simulink model "gmrt_001_intial"  (Simulink 7.5) (continued)
== system gmrt_001_intial == (continued)
      | %else
      | %    set_param(gcb, 'MaskEnables', {'on','on','on','on','on','on','on','on','on','on','on','on','on','on','on','on'});
      | %end
      | 
      | |%specify_mult = get_param(gcb,'specify_mult');
      | %if( strcmp(specify_mult,'off')),
      | %    set_param(gcb, 'MaskEnables', {'on','on','on','on','on','on','on','on','on','on','on','on','on','on','on','off'});
      | %else
      | %    set_param(gcb, 'MaskEnables', {'on','on','on','on','on','on','on','on','on','on','on','on','on','on','on','on'});
      | %end
  pfb_fir_mux3 : Subsystem  AncestorBlock=casper_library_pfbs/pfb_fir_mux  mask(pfb_fir_mux){PFBSize=10, mux_inputs=2, TotalTaps=4, WindowType=hamming, n_inputs=0, MakeBiplex=1, BitWidthIn=12, BitWidthOut=18, CoeffBitWidth=18, CoeffDistMem=0, add_latency=1, mult_latency=2, bram_latency=3, quantization=Round  (unbiased: +/- Inf), fwidth=1, specify_mult=off, mult_spec=[2                                      2]}
      mask prompts: PFBSize "Size of PFB: (2^? pnts)"; mux_inputs "Signals per input (2^?)"; TotalTaps "Total Number of Taps:"; WindowType "Windowing Function:"; n_inputs "Number of Simultaneous Inputs: (2^?)"; MakeBiplex "Make Biplex"; BitWidthIn "Input Bitwidth:"; BitWidthOut "Output Bitwidth:"; CoeffBitWidth "Coefficient Bitwidth:"; CoeffDistMem "Use Distributed Memory for Coeffs"; add_latency "Add Latency"; mult_latency "Mult Latency"; bram_latency "BRAM Latency"; quantization "Quantization Behavior"; fwidth "Bin Width Scaling (normal=1)"; specify_mult "Specify multiplier use (behavioral if not specified)"; mult_spec "Multiplier specification (0=core, 1=embedded, 2=behavioural) (left=1st tap)"
      mask init: same as gmrt_001_intial/pfb_fir_mux1
      settings: AttributesFormatString=taps=4, add_latency=1
      mask Help: eval('xlWeb(''http://casper.berkeley.edu/wiki/Pfb_fir'')')
      mask StyleString: edit,edit,edit,popup(bartlett|barthannwin|blackman|blackmanharris|bohamwin|chebwin|flattopwin|gausswin|hamming|hann|kaiser|nuttallwin|parzenwin|rectwin|tukeywin|triang|userwindow),edit,edit,edit,edit,edit,edit,edit,edit,edit,popup(Truncate|Round  (unbiased: +/- Inf)|Round  (unbiased: Even Values)),edit,checkbox,edit
      mask CallbackString:
      | ||%num_taps = eval(get_param(gcb, 'TotalTaps'));
      | %old_vec = eval(get_param(gcb, 'mult_spec'));
      | 
      | %vec = 2.*ones(1,num_taps);
      | %old_size = length(old_vec);
      | 
      | %if(old_size <= num_taps),
      | %   new_size = old_size;
      | %else,
      | %    new_size = num_taps;
      | %end
      | 
      | %for n = 1:new_size, vec(n) = old_vec(n); end
      | %set_param(gcb, 'mult_spec', mat2str(vec));||||||||||||%num_taps = eval(get_param(gcb, 'TotalTaps'));
      | %old_vec = eval(get_param(gcb, 'mult_spec'));
      | 
      | %vec = 2.*ones(1,num_taps);
      | %old_size = length(old_vec);
      | 
      | %if(old_size <= num_taps),
      | %    new_size = old_size;
      | %else,
      | %    new_size = num_taps;
      | %end
      | 
      | %for n = 1:new_size, vec(n) = old_vec(n); end
      | %set_param(gcb, 'mult_spec', mat2str(vec));|%specify_mult = get_param(gcb,'specify_mult');
      | %if( strcmp(specify_mult,'off')),
      | %    set_param(gcb, 'MaskEnables', {'on','on','on','on','on','on','on','on','on','on','on','on','on','on','on','off'});
      | %else
      | %    set_param(gcb, 'MaskEnables', {'on','on','on','on','on','on','on','on','on','on','on','on','on','on','on','on'});
      | %end
      | 
      | |%specify_mult = get_param(gcb,'specify_mult');
      | %if( strcmp(specify_mult,'off')),
      | %    set_param(gcb, 'MaskEnables', {'on','on','on','on','on','on','on','on','on','on','on','on','on','on','on','off'});
      | %else
      | %    set_param(gcb, 'MaskEnables', {'on','on','on','on','on','on','on','on','on','on','on','on','on','on','on','on'});
      | %end
  pfb_fir_mux4 : Subsystem  AncestorBlock=casper_library_pfbs/pfb_fir_mux  mask(pfb_fir_mux){PFBSize=10, mux_inputs=2, TotalTaps=4, WindowType=hamming, n_inputs=0, MakeBiplex=1, BitWidthIn=12, BitWidthOut=18, CoeffBitWidth=18, CoeffDistMem=0, add_latency=1, mult_latency=2, bram_latency=3, quantization=Round  (unbiased: +/- Inf), fwidth=1, specify_mult=off, mult_spec=[2                                      2]}
      mask prompts: PFBSize "Size of PFB: (2^? pnts)"; mux_inputs "Signals per input (2^?)"; TotalTaps "Total Number of Taps:"; WindowType "Windowing Function:"; n_inputs "Number of Simultaneous Inputs: (2^?)"; MakeBiplex "Make Biplex"; BitWidthIn "Input Bitwidth:"; BitWidthOut "Output Bitwidth:"; CoeffBitWidth "Coefficient Bitwidth:"; CoeffDistMem "Use Distributed Memory for Coeffs"; add_latency "Add Latency"; mult_latency "Mult Latency"; bram_latency "BRAM Latency"; quantization "Quantization Behavior"; fwidth "Bin Width Scaling (normal=1)"; specify_mult "Specify multiplier use (behavioral if not specified)"; mult_spec "Multiplier specification (0=core, 1=embedded, 2=behavioural) (left=1st tap)"
      mask init: same as gmrt_001_intial/pfb_fir_mux1
      settings: AttributesFormatString=taps=4, add_latency=1
      mask Help: eval('xlWeb(''http://casper.berkeley.edu/wiki/Pfb_fir'')')
      mask StyleString: edit,edit,edit,popup(bartlett|barthannwin|blackman|blackmanharris|bohamwin|chebwin|flattopwin|gausswin|hamming|hann|kaiser|nuttallwin|parzenwin|rectwin|tukeywin|triang|userwindow),edit,edit,edit,edit,edit,edit,edit,edit,edit,popup(Truncate|Round  (unbiased: +/- Inf)|Round  (unbiased: Even Values)),edit,checkbox,edit
      mask CallbackString:
      | ||%num_taps = eval(get_param(gcb, 'TotalTaps'));
      | %old_vec = eval(get_param(gcb, 'mult_spec'));
      | 
      | %vec = 2.*ones(1,num_taps);
      | %old_size = length(old_vec);
      | 
      | %if(old_size <= num_taps),
      | %   new_size = old_size;
      | %else,
      | %    new_size = num_taps;
      | %end
      | 
      | %for n = 1:new_size, vec(n) = old_vec(n); end
      | %set_param(gcb, 'mult_spec', mat2str(vec));||||||||||||%num_taps = eval(get_param(gcb, 'TotalTaps'));
      | %old_vec = eval(get_param(gcb, 'mult_spec'));
      | 
      | %vec = 2.*ones(1,num_taps);
      | %old_size = length(old_vec);
      | 
      | %if(old_size <= num_taps),
      | %    new_size = old_size;
      | %else,
      | %    new_size = num_taps;
      | %end
      | 
      | %for n = 1:new_size, vec(n) = old_vec(n); end
      | %set_param(gcb, 'mult_spec', mat2str(vec));|%specify_mult = get_param(gcb,'specify_mult');
      | %if( strcmp(specify_mult,'off')),
      | %    set_param(gcb, 'MaskEnables', {'on','on','on','on','on','on','on','on','on','on','on','on','on','on','on','off'});
      | %else
      | %    set_param(gcb, 'MaskEnables', {'on','on','on','on','on','on','on','on','on','on','on','on','on','on','on','on'});
      | %end
      | 
      | |%specify_mult = get_param(gcb,'specify_mult');
      | %if( strcmp(specify_mult,'off')),
      | %    set_param(gcb, 'MaskEnables', {'on','on','on','on','on','on','on','on','on','on','on','on','on','on','on','off'});
      | %else
      | %    set_param(gcb, 'MaskEnables', {'on','on','on','on','on','on','on','on','on','on','on','on','on','on','on','on'});
      | %end
  phase_EQ1 : Subsystem  mask{N=10+5 - 2, bram_lat=2, mult_lat=3, add_lat=2, chans_per_coeff=2}
      mask prompts: N "number of coefficients (2^?)"; bram_lat "bram latency"; mult_lat "multiplier latency"; add_lat "adder latency"; chans_per_coeff "channels per coeff (2^?)"
  pipeline : Subsystem  AncestorBlock=casper_library/Delays/pipeline  mask(pipeline){latency=1}
      mask help:
      | An explicitly laid-out delay line to avoid mapping to SRL16s
      | for use in pipelining to help achieve timing closure.
      mask init:
      | pipeline_init(gcb, 'latency', latency);
      mask Display:
      | port_label('input', 1, 'd');
      | port_label('output', 1, 'q');
      | disp(['Z^-', num2str(latency)]);
  pipeline1 : Subsystem  AncestorBlock=casper_library/Delays/pipeline  mask(pipeline){latency=2}
      mask help:
      | An explicitly laid-out delay line to avoid mapping to SRL16s
      | for use in pipelining to help achieve timing closure.
      mask init:
      | pipeline_init(gcb, 'latency', latency);
      mask Display:
      | port_label('input', 1, 'd');
      | port_label('output', 1, 'q');
      | disp(['Z^-', num2str(latency)]);
  pipeline10 : Subsystem  AncestorBlock=casper_library/Delays/pipeline  mask(pipeline){latency=1}
      mask help:
      | An explicitly laid-out delay line to avoid mapping to SRL16s
      | for use in pipelining to help achieve timing closure.
      mask init:
      | pipeline_init(gcb, 'latency', latency);
      mask Display:
      | port_label('input', 1, 'd');
      | port_label('output', 1, 'q');
      | disp(['Z^-', num2str(latency)]);
  pipeline11 : Subsystem  AncestorBlock=casper_library/Delays/pipeline  mask(pipeline){latency=1}
      mask help:
      | An explicitly laid-out delay line to avoid mapping to SRL16s
      | for use in pipelining to help achieve timing closure.
      mask init:
      | pipeline_init(gcb, 'latency', latency);
      mask Display:
      | port_label('input', 1, 'd');
      | port_label('output', 1, 'q');
      | disp(['Z^-', num2str(latency)]);
  pipeline12 : Subsystem  AncestorBlock=casper_library/Delays/pipeline  mask(pipeline){latency=1}
      mask help:
      | An explicitly laid-out delay line to avoid mapping to SRL16s
      | for use in pipelining to help achieve timing closure.
      mask init:
      | pipeline_init(gcb, 'latency', latency);
      mask Display:
      | port_label('input', 1, 'd');
      | port_label('output', 1, 'q');
      | disp(['Z^-', num2str(latency)]);
  pipeline13 : Subsystem  AncestorBlock=casper_library/Delays/pipeline  mask(pipeline){latency=1}
      mask help:
      | An explicitly laid-out delay line to avoid mapping to SRL16s
      | for use in pipelining to help achieve timing closure.
      mask init:
      | pipeline_init(gcb, 'latency', latency);
      mask Display:
      | port_label('input', 1, 'd');
      | port_label('output', 1, 'q');
      | disp(['Z^-', num2str(latency)]);
  pipeline14 : Subsystem  AncestorBlock=casper_library/Delays/pipeline  mask(pipeline){latency=1}
      mask help:
      | An explicitly laid-out delay line to avoid mapping to SRL16s
      | for use in pipelining to help achieve timing closure.
      mask init:
      | pipeline_init(gcb, 'latency', latency);
      mask Display:
      | port_label('input', 1, 'd');
      | port_label('output', 1, 'q');
      | disp(['Z^-', num2str(latency)]);
  pipeline15 : Subsystem  AncestorBlock=casper_library/Delays/pipeline  mask(pipeline){latency=1}
      mask help:
      | An explicitly laid-out delay line to avoid mapping to SRL16s
      | for use in pipelining to help achieve timing closure.
      mask init:
      | pipeline_init(gcb, 'latency', latency);
      mask Display:
      | port_label('input', 1, 'd');
      | port_label('output', 1, 'q');
      | disp(['Z^-', num2str(latency)]);
  pipeline16 : Subsystem  AncestorBlock=casper_library/Delays/pipeline  mask(pipeline){latency=1}
      mask help:
      | An explicitly laid-out delay line to avoid mapping to SRL16s
      | for use in pipelining to help achieve timing closure.
      mask init:
      | pipeline_init(gcb, 'latency', latency);
      mask Display:
      | port_label('input', 1, 'd');
      | port_label('output', 1, 'q');
      | disp(['Z^-', num2str(latency)]);
  pipeline17 : Subsystem  AncestorBlock=casper_library/Delays/pipeline  mask(pipeline){latency=2}
      mask help:
      | An explicitly laid-out delay line to avoid mapping to SRL16s
      | for use in pipelining to help achieve timing closure.
      mask init:
      | pipeline_init(gcb, 'latency', latency);
      mask Display:
      | port_label('input', 1, 'd');
      | port_label('output', 1, 'q');
      | disp(['Z^-', num2str(latency)]);
  pipeline24 : Subsystem  AncestorBlock=casper_library/Delays/pipeline  mask(pipeline){latency=1}
      mask help:
      | An explicitly laid-out delay line to avoid mapping to SRL16s
      | for use in pipelining to help achieve timing closure.
      mask init:
      | pipeline_init(gcb, 'latency', latency);
      mask Display:
      | port_label('input', 1, 'd');
      | port_label('output', 1, 'q');
      | disp(['Z^-', num2str(latency)]);
  pipeline25 : Subsystem  AncestorBlock=casper_library/Delays/pipeline  mask(pipeline){latency=1}
      mask help:
      | An explicitly laid-out delay line to avoid mapping to SRL16s
      | for use in pipelining to help achieve timing closure.
      mask init:
      | pipeline_init(gcb, 'latency', latency);
      mask Display:
      | port_label('input', 1, 'd');
      | port_label('output', 1, 'q');
      | disp(['Z^-', num2str(latency)]);
  pipeline26 : Subsystem  AncestorBlock=casper_library/Delays/pipeline  mask(pipeline){latency=1}
      mask help:
      | An explicitly laid-out delay line to avoid mapping to SRL16s
      | for use in pipelining to help achieve timing closure.
      mask init:
      | pipeline_init(gcb, 'latency', latency);
      mask Display:
      | port_label('input', 1, 'd');
      | port_label('output', 1, 'q');
      | disp(['Z^-', num2str(latency)]);
  pipeline27 : Subsystem  AncestorBlock=casper_library/Delays/pipeline  mask(pipeline){latency=1}
      mask help:
      | An explicitly laid-out delay line to avoid mapping to SRL16s
      | for use in pipelining to help achieve timing closure.
      mask init:
      | pipeline_init(gcb, 'latency', latency);
      mask Display:
      | port_label('input', 1, 'd');
      | port_label('output', 1, 'q');
      | disp(['Z^-', num2str(latency)]);
  pipeline28 : Subsystem  AncestorBlock=casper_library/Delays/pipeline  mask(pipeline){latency=1}
      mask help:
      | An explicitly laid-out delay line to avoid mapping to SRL16s
      | for use in pipelining to help achieve timing closure.
      mask init:
      | pipeline_init(gcb, 'latency', latency);
      mask Display:
      | port_label('input', 1, 'd');
      | port_label('output', 1, 'q');
      | disp(['Z^-', num2str(latency)]);
  pipeline29 : Subsystem  AncestorBlock=casper_library/Delays/pipeline  mask(pipeline){latency=1}
      mask help:
      | An explicitly laid-out delay line to avoid mapping to SRL16s
      | for use in pipelining to help achieve timing closure.
      mask init:
      | pipeline_init(gcb, 'latency', latency);
      mask Display:
      | port_label('input', 1, 'd');
      | port_label('output', 1, 'q');
      | disp(['Z^-', num2str(latency)]);
  pipeline30 : Subsystem  AncestorBlock=casper_library/Delays/pipeline  mask(pipeline){latency=1}
      mask help:
      | An explicitly laid-out delay line to avoid mapping to SRL16s
      | for use in pipelining to help achieve timing closure.
      mask init:
      | pipeline_init(gcb, 'latency', latency);
      mask Display:
      | port_label('input', 1, 'd');
      | port_label('output', 1, 'q');
      | disp(['Z^-', num2str(latency)]);
  pipeline31 : Subsystem  AncestorBlock=casper_library/Delays/pipeline  mask(pipeline){latency=1}
      mask help:
      | An explicitly laid-out delay line to avoid mapping to SRL16s
      | for use in pipelining to help achieve timing closure.
      mask init:
      | pipeline_init(gcb, 'latency', latency);
      mask Display:
      | port_label('input', 1, 'd');
      | port_label('output', 1, 'q');
      | disp(['Z^-', num2str(latency)]);
  pipeline32 : Subsystem  AncestorBlock=casper_library/Delays/pipeline  mask(pipeline){latency=1}
      mask help:
      | An explicitly laid-out delay line to avoid mapping to SRL16s
      | for use in pipelining to help achieve timing closure.
      mask init:
      | pipeline_init(gcb, 'latency', latency);
      mask Display:
      | port_label('input', 1, 'd');
      | port_label('output', 1, 'q');
      | disp(['Z^-', num2str(latency)]);
  pipeline33 : Subsystem  AncestorBlock=casper_library/Delays/pipeline  mask(pipeline){latency=1}
      mask help:
      | An explicitly laid-out delay line to avoid mapping to SRL16s
      | for use in pipelining to help achieve timing closure.
      mask init:
      | pipeline_init(gcb, 'latency', latency);
      mask Display:
      | port_label('input', 1, 'd');
      | port_label('output', 1, 'q');
      | disp(['Z^-', num2str(latency)]);
  pipeline34 : Subsystem  AncestorBlock=casper_library/Delays/pipeline  mask(pipeline){latency=1}
      mask help:
      | An explicitly laid-out delay line to avoid mapping to SRL16s
      | for use in pipelining to help achieve timing closure.
      mask init:
      | pipeline_init(gcb, 'latency', latency);
      mask Display:
      | port_label('input', 1, 'd');
      | port_label('output', 1, 'q');
      | disp(['Z^-', num2str(latency)]);
  pipeline35 : Subsystem  AncestorBlock=casper_library/Delays/pipeline  mask(pipeline){latency=1}
      mask help:
      | An explicitly laid-out delay line to avoid mapping to SRL16s
      | for use in pipelining to help achieve timing closure.
      mask init:
      | pipeline_init(gcb, 'latency', latency);
      mask Display:
      | port_label('input', 1, 'd');
      | port_label('output', 1, 'q');
      | disp(['Z^-', num2str(latency)]);
  pipeline4 : Subsystem  AncestorBlock=casper_library/Delays/pipeline  mask(pipeline){latency=1}
      mask help:
      | An explicitly laid-out delay line to avoid mapping to SRL16s
      | for use in pipelining to help achieve timing closure.
      mask init:
      | pipeline_init(gcb, 'latency', latency);
      mask Display:
      | port_label('input', 1, 'd');
      | port_label('output', 1, 'q');
      | disp(['Z^-', num2str(latency)]);
  power : casper_library_misc/power  SystemSampleTime=-1  BitWidth=18  add_latency=0  mult_latency=0
  power1 : casper_library_misc/power  SystemSampleTime=-1  BitWidth=18  add_latency=0  mult_latency=0
  pulse_ext : casper_library_misc/pulse_ext  SystemSampleTime=-1  pulse_len=2^26
  pulse_ext1 : casper_library_misc/pulse_ext  SystemSampleTime=-1  pulse_len=2^25
  q_snap : Subsystem
  quant1 : Subsystem
  quant2 : Subsystem
  quant3 : Subsystem
  quant4 : Subsystem
  ri_to_c{,1..7} (8 alike) : casper_library_misc/ri_to_c  SystemSampleTime=-1
  sim_sync1 : ox_lib/sim_sync  SystemSampleTime=-1  delay=9
  sim_sync3 : ox_lib/sim_sync  SystemSampleTime=-1  delay=10
  sim_val : Subsystem  AncestorBlock=ox_lib/sim_sync  mask{val=2^11-1, bits=11}
      mask prompts: val "value"
  snap_ctrl : xps_library/software register  Tag=xps:sw_reg  SystemSampleTime=-1  io_dir=From Processor  arith_type=Unsigned  bitwidth=32  bin_pt=0  sample_period=1  gw_name=gmrt_001_intial_FFTT_snap_ctrl_user_data_out
  square_transposer{,1..8} (9 alike) : casper_library_reorder/square_transposer  SystemSampleTime=-1  n_inputs=1
      settings: AttributesFormatString=n_inputs=1
  sync_gen : Subsystem
  sync_gpio : xps_library/gpio  Tag=xps:gpio  SystemSampleTime=-1  io_group=ROACH:gpioa  io_dir=in  arith_type=Boolean  bitwidth=1  bin_pt=0  bit_index=6  sample_period=1  use_single_ended=off  use_ddr=off  reg_iob=on  reg_clk_phase=0  termination=Pulldown
  sync_gpio_io_sel : xps_library/gpio  Tag=xps:gpio  SystemSampleTime=-1  io_group=ROACH:gpioa_oe_n  io_dir=out  arith_type=Boolean  bitwidth=1  bin_pt=0  bit_index=0  sample_period=1  use_single_ended=off  use_ddr=off  reg_iob=on  reg_clk_phase=0  termination=Pulldown
  sync_led0 : xps_library/gpio  Tag=xps:gpio  SystemSampleTime=-1  io_group=ROACH:led  io_dir=out  arith_type=Boolean  bitwidth=1  bin_pt=0  bit_index=0  sample_period=1  use_single_ended=off  use_ddr=off  reg_iob=on  reg_clk_phase=0  termination=Pulldown
  sync_led1 : xps_library/gpio  Tag=xps:gpio  SystemSampleTime=-1  io_group=ROACH:led  io_dir=out  arith_type=Boolean  bitwidth=1  bin_pt=0  bit_index=1  sample_period=1  use_single_ended=off  use_ddr=off  reg_iob=on  reg_clk_phase=0  termination=Pulldown
  uncram : Subsystem  AncestorBlock=gavrt_library/uncram  mask(uncram){num_slice=8, slice_width=1, bin_pt=0, arith_type=0}
      mask prompts: num_slice "Number of slices"; slice_width "Slice Width"; bin_pt "Output Binary Point"; arith_type "Output Arithmetic Type (0=Unsigned, 1=Signed)"
      mask help: Takes a concatenated input and slices it up into even pieces and reinterprets them as signed fixed point numbers with a given binary point.
      mask init:
      | uncram_init(gcb, ...
      |     'num_slice', num_slice, ...
      |     'slice_width', slice_width, ...
      |     'bin_pt', bin_pt, ...
      |     'arith_type', arith_type);
      settings: AttributesFormatString=UFix_1_0
  x64_adc : Subsystem  Tag=xps:x64_adc  AncestorBlock=ox_lib/x64_adc  mask(x64_adc){adc_clk_rate=50, spi=off, ctrl_gpio=GPIO_B}
      mask prompts: adc_clk_rate "ADC clock rate (MHz)"; spi "Include SPI interface?"; ctrl_gpio "GPIO bank to use for control signals?"
      mask help: The ADC block converts analog inputs to digital outputs. Every clock cycle, the inputs are sampled and digitized to 8 bit binary point numbers in the range of [-1, 1) and are then output by the adc.
      mask init:
      | x64_adc_mask(gcb);
      mask Help: eval('xlWeb([getenv(''MLIB_ROOT''), ''\doc\html\sys_adc\sys_adc.html''])')
      mask StyleString: edit,checkbox,popup(GPIO_A|GPIO_B)
  wires:
  Constant20/1 -> ctrl_sw/1
  ctrl_sw/1 -> Slice/1, Slice4/1, Slice2/1, Slice12/1, Slice11/1, Slice5/1, Slice10/1, Slice13/1
  Slice4/1 -> pipeline15/1(d)
  adc_spi_ctrl/1 -> Slice3/1
  ri_to_c1/1 -> pfb_fir_mux1/3(pol2_in1)
  mux_32_streams2/1(sy nc_out) -> Delay10/1
  Slice3/1 -> x64_adc/18(adc_rst)
  ri_to_c/1 -> pfb_fir_mux1/2(pol1_in1)
  x64_adc/17(chan_sync) -> Delay5/1
  [adc0_3i_in] -> adc_sw/1(in0)
  [adc0_3q_in] -> adc_sw/2(in1)
  [adc_chan_sync] -> Slice1/1
  Slice1/1 -> adc_sw/9(chan_sync)
  Slice/1 -> pipeline16/1(d)
  Slice2/1 -> pipeline14/1(d)
  sync_gen/1(sync) -> sim_sync3/1
  [arm_rst] -> sync_gen/2(arm)
  sync_gen/3(armed) -> [armed]
  Logical7/1 -> edge_detect/1
  sync_gpio/1 -> Logical7/1, edge_detect1/1
  [sync_rst] -> Logical7/2
  sim_sync3/1 -> adc_sync_align/1(sync)
  adc_sync_align/1(sync_out) -> [sync_gen], pulse_ext1/1
  sync_gen/2(mrst) -> sim_sync1/1
  adc_sync_align1/1(sync_out) -> [mrst]
  [adc_chan_sync] -> Slice7/1
  [amp_EQ_sync_out] -> Delay2/1
  Delay2/1 -> [quant_sync]
  quant4/2(of) -> [quant_of0]
  Concat5/1 -> pipeline/1(d)
  Slice12/1 -> pipeline13/1(d)
  Slice7/1 -> adc_sync_align/2(adc_sync), adc_sync_align1/2(adc_sync)
  adc_sim_sync/1(Out1) -> x64_adc/17(sim_sync)
  [adc_chan_sync] -> uncram/1(In)
  uncram/1(Out1) -> Logical1/1
  uncram/2(Out2) -> Logical1/2
  uncram/3(Out3) -> Logical1/3
  uncram/4(Out4) -> Logical1/4
  uncram/5(Out5) -> Logical1/5
  uncram/6(Out6) -> Logical1/6
  uncram/7(Out7) -> Logical1/7
  uncram/8(Out8) -> Logical1/8
  Logical1/1 -> Convert/1
  [sync_gen] -> Delay/1
  Delay/1 -> Logical2/1
  [adc_chan_sync] -> Slice9/1
  Slice9/1 -> Inverter/1
  Inverter/1 -> Logical2/2
  Logical2/1 -> Convert1/1
  Delay3/1 -> adc_sync_align1/1(sync)
  Concat6/1 -> adc_sync_test/1
  sim_sync1/1 -> Delay3/1
  Convert/1 -> ext21/1(in)
  Convert1/1 -> ext22/1(in)
  Slice11/1 -> pipeline12/1(d)
  x64_adc/1(dout0) -> Mux13/2, Mux13/3, Mux6/3, Mux7/3, Mux4/3, Mux5/3, Mux2/3, Mux3/3, Mux1/3
  x64_adc/2(dout1) -> Mux1/2
  x64_adc/3(dout2) -> Mux3/2
  x64_adc/4(dout3) -> Mux2/2
  x64_adc/5(dout4) -> Mux5/2
  x64_adc/6(dout5) -> Mux4/2
  x64_adc/7(dout6) -> Mux7/2
  x64_adc/8(dout7) -> Mux6/2
  [debug_sigs] -> Mux7/1, Mux6/1, Mux12/1, Mux11/1, Mux15/1, Mux14/1, Mux17/1, Mux16/1, Mux18/1, Mux19/1, Mux4/1, Mux5/1, Mux2/1, Mux3/1, Mux1/1, Mux13/1
  [amp_0_7h_i_out] -> quant4/1(din)
  ext22/1(out) -> Concat6/2
  ext21/1(out) -> Concat6/1
  [adc4_7i_in] -> adc_sw/3(in2)
  [adc4_7q_in] -> adc_sw/4(in3)
  [adc8_11i_in] -> adc_sw/5(in4)
  [adc8_11q_in] -> adc_sw/6(in5)
  [adc12_15i_in] -> adc_sw/7(in6)
  [adc12_15q_in] -> adc_sw/8(in7)
  [fft_of_0] -> Logical/1
  [fft_of_1] -> Logical/2
  Logical/1 -> ext1/1(in)
  pipeline/1(q) -> of_reg/1
  quant4/1(dout) -> [quant_h_high]
  adc_sw/1(adc_tv_out) -> [adc_tv]
  Conant/1 -> sync_gpio_io_sel/1
  edge_detect/1 -> sync_gen/1(rst)
  pipeline1/1(q) -> sync_led0/1
  edge_detect1/1 -> pulse_ext/1
  pulse_ext/1 -> pipeline1/1(d)
  pulse_ext1/1 -> pipeline17/1(d)
  phase_EQ1/1(sync_out) -> [phase_EQ_sync_out]
  [fir_sync_out] -> Delay6/1
  fft_biplex_real_2x1/1(sync_out) -> Gateway Out5/1, Delay9/1
  [fir0_3h_out] -> pipeline27/1(d)
  [fir4_7h_out] -> pipeline26/1(d)
  [fir0_3v_out] -> pipeline25/1(d)
  [fir4_7v_out] -> pipeline24/1(d)
  fft_biplex_real_2x2/4(of) -> Delay7/1
  fft_biplex_real_2x2/2(pol02_out) -> pipeline30/1(d)
  fft_biplex_real_2x2/3(pol13_out) -> pipeline29/1(d)
  [phase_EQ_sync_out] -> amp_EQ1/1(sync_in)
  amp_EQ1/3(A_of) -> [amp_of_0]
  amp_EQ1/5(B_of) -> [amp_of_1]
  amp_EQ1/7(C_of) -> [amp_of_2]
  amp_EQ1/9(D_of) -> [amp_of_3]
  amp_EQ1/1(sync_out) -> [amp_EQ_sync_out]
  amp_EQ1/2(doutA) -> [amp_0_7h_i_out]
  amp_EQ1/4(doutB) -> [amp_0_7h_q_out]
  amp_EQ1/6(doutC) -> [amp_0_7v_i_out]
  amp_EQ1/8(doutD) -> [amp_0_7v_q_out]
  [sb_sync_out] -> phase_EQ1/1(sync_in)
  [sb_high_h] -> phase_EQ1/2(dinA)
  [sb_low_h] -> phase_EQ1/3(dinB)
  [sb_high_v] -> phase_EQ1/4(dinC)
  [sb_low_v] -> phase_EQ1/5(dinD)
  phase_EQ1/2(doutA) -> [phase_EQ_h_i]
  phase_EQ1/3(doutB) -> [phase_EQ_h_q]
  phase_EQ1/4(doutC) -> [phase_EQ_v_i]
  phase_EQ1/5(doutD) -> [phase_EQ_v_q]
  quant1/2(of) -> [quant_of1]
  [amp_0_7h_q_out] -> quant1/1(din)
  quant1/1(dout) -> [quant_h_low]
  quant2/2(of) -> [quant_of2]
  [amp_0_7v_i_out] -> quant2/1(din)
  quant2/1(dout) -> [quant_v_high]
  quant3/2(of) -> [quant_of3]
  [amp_0_7v_q_out] -> quant3/1(din)
  quant3/1(dout) -> [quant_v_low]
  [quant] -> i_snap/9(quant0)
  [fft_sync_out] -> i_snap/10(fft_sync)
  [amp_EQ_sync_out] -> i_snap/11(amp_sync)
  [phase_EQ_sync_out] -> i_snap/12(phase_sync)
  [quant_sync] -> i_snap/14(quant_sync)
  [fft_0_7h_i_out] -> i_snap/1(fft0)
  [fft_0_7v_i_out] -> i_snap/2(fft1)
  [amp_0_7h_i_out] -> i_snap/3(amp0)
  [amp_0_7v_i_out] -> i_snap/4(amp1)
  [phase_EQ_h_i] -> i_snap/5(phase0)
  [phase_EQ_v_i] -> i_snap/6(phase1)
  Logical3/1 -> ext2/1(in)
  [amp_of_0] -> Logical3/1
  [amp_of_1] -> Logical3/2
  [amp_of_2] -> Logical3/3
  [amp_of_3] -> Logical3/4
  [sb_of] -> ext3/1(in)
  Mux13/1 -> coarse_delay/1(din0)
  Mux1/1 -> coarse_delay/2(din1)
  Mux3/1 -> coarse_delay/3(din2)
  Mux2/1 -> coarse_delay/4(din3)
  Mux5/1 -> coarse_delay/5(din4)
  Mux4/1 -> coarse_delay/6(din5)
  Mux7/1 -> coarse_delay/7(din6)
  Mux6/1 -> coarse_delay/8(din7)
  c_to_ri/1 -> fft_biplex_real_2x2/3(pol0)
  c_to_ri/2 -> fft_biplex_real_2x2/4(pol1)
  c_to_ri1/1 -> fft_biplex_real_2x2/5(pol2)
  c_to_ri1/2 -> fft_biplex_real_2x2/6(pol3)
  [mux_sync_out] -> fft_biplex_real_2x2/1(sync)
  [shift] -> fft_biplex_real_2x2/2(shift)
  mux_32_streams2/4(Out2) -> pipeline34/1(d)
  mux_32_streams2/5(Out3) -> pipeline35/1(d)
  c_to_ri2/1 -> fft_biplex_real_2x1/3(pol0), Gateway Out/1
  c_to_ri2/2 -> fft_biplex_real_2x1/4(pol1)
  c_to_ri3/1 -> fft_biplex_real_2x1/5(pol2)
  c_to_ri3/2 -> fft_biplex_real_2x1/6(pol3)
  [mux_sync_out] -> fft_biplex_real_2x1/1(sync), Gateway Out3/1
  [shift] -> fft_biplex_real_2x1/2(shift)
  fft_biplex_real_2x1/2(pol02_out) -> power/1, pipeline32/1(d)
  fft_biplex_real_2x1/3(pol13_out) -> power1/1, pipeline31/1(d)
  fft_biplex_real_2x1/4(of) -> Gateway Out7/1, Delay8/1
  mux_32_streams2/2(Out0) -> pipeline28/1(d)
  mux_32_streams2/3(Out1) -> pipeline33/1(d)
  [sb_high_h] -> i_snap/7(high0)
  [sb_high_v] -> i_snap/8(high1)
  [sb_sync_out] -> i_snap/13(subband_sync)
  Constant13/1 -> snap_ctrl/1
  [fft_0_7h_q_out] -> q_snap/1(fft0)
  [fft_0_7v_q_out] -> q_snap/2(fft1)
  [amp_0_7h_q_out] -> q_snap/3(amp0)
  [amp_0_7v_q_out] -> q_snap/4(amp1)
  [phase_EQ_h_q] -> q_snap/5(phase0)
  [phase_EQ_v_q] -> q_snap/6(phase1)
  [sb_low_h] -> q_snap/7(high0)
  [sb_low_v] -> q_snap/8(high1)
  [transpose_out] -> q_snap/9(quant0)
  [fft_sync_out] -> q_snap/10(fft_sync)
  [amp_EQ_sync_out] -> q_snap/11(amp_sync)
  [phase_EQ_sync_out] -> q_snap/12(phase_sync)
  [sb_sync_out] -> q_snap/13(subband_sync)
  [transpose_sync_out] -> q_snap/14(quant_sync)
  snap_ctrl/1 -> i_snap/15(ctrl_reg), q_snap/15(ctrl_reg)
  Slice5/1 -> pipeline11/1(d)
  [phase_EQ_h_i] -> amp_EQ1/2(dinA)
  [phase_EQ_h_q] -> amp_EQ1/3(dinB)
  [phase_EQ_v_i] -> amp_EQ1/4(dinC)
  [phase_EQ_v_q] -> amp_EQ1/5(dinD)
  Slice10/1 -> pipeline4/1(d)
  Slice13/1 -> pipeline10/1(d)
  phase_EQ1/6(doutAof) -> [phase_of_0]
  phase_EQ1/7(doutBof) -> [phase_of_1]
  phase_EQ1/8(doutCof) -> [phase_of_2]
  phase_EQ1/9(doutDof) -> [phase_of_3]
  [phase_of_0] -> Logical4/1
  [phase_of_1] -> Logical4/2
  [phase_of_2] -> Logical4/3
  [phase_of_3] -> Logical4/4
  pipeline10/1(q) -> edge_detect2/1
  pipeline4/1(q) -> edge_detect3/1
  pipeline12/1(q) -> [debug_sigs]
  pipeline11/1(q) -> [subband_sel]
  pipeline13/1(q) -> [xaui_rcv_rst]
  pipeline14/1(q) -> [sync_rst]
  pipeline15/1(q) -> [arm_rst]
  pipeline16/1(q) -> sim_val/1(sync_in)
  pipeline17/1(q) -> sync_led1/1
  edge_detect2/1 -> [mcnt_rst]
  edge_detect3/1 -> [acc_ctr_rst]
  ext3/1(out) -> Concat5/6
  ext2/1(out) -> Concat5/5
  ext1/1(out) -> Concat5/4
  [fftt_of] -> ext4/1(in)
  ext4/1(out) -> Concat5/3
  Logical4/1 -> ext5/1(in)
  ext5/1(out) -> Concat5/2
  ext6/1(out) -> Concat5/1
  [x_s_clash] -> ext6/1(in)
  phase_EQ1/10(3_clk_warn) -> amp_EQ1/6(3_clk_warn_in)
  Delay5/1 -> [adc_chan_sync]
  Product/1 -> x64_adc/1(sim0)
  Counter Free-Running/1 -> Product/2
  Constant/1 -> Product/1
  Gateway Out/1 -> Scope/1
  Gateway Out3/1 -> Scope/2
  Gateway Out5/1 -> Scope/3
  Gateway Out4/1 -> Scope/4
  Gateway Out6/1 -> Scope/5
  power/1 -> Gateway Out4/1
  power1/1 -> Gateway Out6/1
  Gateway Out7/1 -> Scope/6, Scope/7, Scope/8
  sim_val/1(sync_out) -> [shift]
  [quant_v_low] -> Concat/5
  [quant_h_low] -> Concat/4
  [quant_v_high] -> Concat/3
  [quant_h_high] -> Concat/2
  Constant2/1 -> Concat/1
  pipeline24/1(q) -> mux_32_streams2/5(in3)
  pipeline25/1(q) -> mux_32_streams2/4(in2)
  pipeline26/1(q) -> mux_32_streams2/3(in1)
  pipeline27/1(q) -> mux_32_streams2/2(in0)
  Delay6/1 -> mux_32_streams2/1(sync)
  Delay7/1 -> [fft_of_1]
  pipeline29/1(q) -> [fft_0_7v_q_out]
  pipeline30/1(q) -> [fft_0_7v_i_out]
  pipeline31/1(q) -> [fft_0_7h_q_out]
  pipeline32/1(q) -> [fft_0_7h_i_out]
  Delay8/1 -> [fft_of_0]
  Delay9/1 -> [fft_sync_out]
  pipeline28/1(q) -> c_to_ri2/1
  pipeline33/1(q) -> c_to_ri3/1
  pipeline34/1(q) -> c_to_ri/1
  pipeline35/1(q) -> c_to_ri1/1
  Delay10/1 -> [mux_sync_out]
  x64_adc/9(dout8) -> Mux11/3, Mux15/3, Mux14/3, Mux17/3, Mux16/3, Mux19/3, Mux18/3, Mux12/3, Mux12/2
  x64_adc/10(dout9) -> Mux11/2
  x64_adc/11(dout10) -> Mux15/2
  x64_adc/12(dout11) -> Mux14/2
  x64_adc/13(dout12) -> Mux17/2
  x64_adc/14(dout13) -> Mux16/2
  x64_adc/15(dout14) -> Mux19/2
  x64_adc/16(dout15) -> Mux18/2
  Mux12/1 -> coarse_delay/9(din8)
  Mux11/1 -> coarse_delay/10(din9)
  Mux15/1 -> coarse_delay/11(din10)
  Mux14/1 -> coarse_delay/12(din11)
  Mux17/1 -> coarse_delay/13(din12)
  Mux16/1 -> coarse_delay/14(din13)
  Mux19/1 -> coarse_delay/15(din14)
  Mux18/1 -> coarse_delay/16(din15)
  coarse_delay/1(dout0) -> [adc0_3_in]
  coarse_delay/2(dout1) -> [adc4_7_in]
  coarse_delay/3(dout2) -> [adc8_11_in]
  coarse_delay/4(dout3) -> [adc12_15_in]
  coarse_delay/5(dout4) -> [adc16_19_in]
  coarse_delay/6(dout5) -> [adc20_23_in]
  coarse_delay/7(dout6) -> [adc24_27_in]
  coarse_delay/8(dout7) -> [adc28_31_in]
  coarse_delay/9(dout8) -> [adc32_35_in]
  coarse_delay/10(dout9) -> [adc36_39_in]
  coarse_delay/11(dout10) -> [adc40_43_in]
  coarse_delay/12(dout11) -> [adc44_47_in]
  coarse_delay/13(dout12) -> [adc48_51_in]
  coarse_delay/14(dout13) -> [adc52_55_in]
  coarse_delay/15(dout14) -> [adc56_59_in]
  coarse_delay/16(dout15) -> [adc60_63_in]
  [sync_gen] -> square_transposer/1, square_transposer1/1
  square_transposer/1 -> pfb_fir_mux1/1(sync)
  square_transposer/2 -> ri_to_c/1
  square_transposer/3 -> ri_to_c/2
  square_transposer1/2 -> ri_to_c1/1
  square_transposer1/3 -> ri_to_c1/2
  [adc0_3_in] -> square_transposer/2
  [adc4_7_in] -> square_transposer/3
  [adc8_11_in] -> square_transposer1/2
  [adc12_15_in] -> square_transposer1/3
  ri_to_c3/1 -> pfb_fir_mux2/3(pol2_in1)
  ri_to_c2/1 -> pfb_fir_mux2/2(pol1_in1)
  pfb_fir_mux2/2(pol1_out1) -> [fir0_3h_out]
  pfb_fir_mux2/3(pol2_out1) -> [fir4_7h_out]
  [sync_gen] -> square_transposer3/1, square_transposer2/1
  square_transposer2/1 -> pfb_fir_mux2/1(sync)
  square_transposer2/2 -> ri_to_c2/1
  square_transposer2/3 -> ri_to_c2/2
  square_transposer3/2 -> ri_to_c3/1
  square_transposer3/3 -> ri_to_c3/2
  [adc16_19_in] -> square_transposer2/2
  [adc20_23_in] -> square_transposer2/3
  [adc24_27_in] -> square_transposer3/2
  [adc28_31_in] -> square_transposer3/3
  ri_to_c5/1 -> pfb_fir_mux3/3(pol2_in1)
  ri_to_c4/1 -> pfb_fir_mux3/2(pol1_in1)
  pfb_fir_mux3/2(pol1_out1) -> [fir0_3h_out]
  pfb_fir_mux3/3(pol2_out1) -> [fir4_7h_out]
  [sync_gen] -> square_transposer4/1, square_transposer5/1
  square_transposer4/1 -> pfb_fir_mux3/1(sync)
  square_transposer4/2 -> ri_to_c4/1
  square_transposer4/3 -> ri_to_c4/2
  square_transposer5/2 -> ri_to_c5/1
  square_transposer5/3 -> ri_to_c5/2
  [adc32_35_in] -> square_transposer4/2
  [adc36_39_in] -> square_transposer4/3
  [adc40_43_in] -> square_transposer5/2
  [adc44_47_in] -> square_transposer5/3
  ri_to_c7/1 -> pfb_fir_mux4/3(pol2_in1)
  ri_to_c6/1 -> pfb_fir_mux4/2(pol1_in1)
  pfb_fir_mux4/2(pol1_out1) -> [fir0_3h_out]
  pfb_fir_mux4/3(pol2_out1) -> [fir4_7h_out]
  [sync_gen] -> square_transposer7/1, square_transposer6/1
  square_transposer6/1 -> pfb_fir_mux4/1(sync)
  square_transposer6/2 -> ri_to_c6/1
  square_transposer6/3 -> ri_to_c6/2
  square_transposer7/2 -> ri_to_c7/1
  square_transposer7/3 -> ri_to_c7/2
  [adc48_51_in] -> square_transposer6/2
  [adc52_55_in] -> square_transposer6/3
  [adc56_59_in] -> square_transposer7/2
  [adc60_63_in] -> square_transposer7/3
  pfb_fir_mux1/1(sync_out) -> square_transposer8/1
  pfb_fir_mux1/2(pol1_out1) -> square_transposer8/2
  pfb_fir_mux1/3(pol2_out1) -> square_transposer8/3
  square_transposer8/2 -> [fir0_3l_out]
  square_transposer8/3 -> [fir0_3h_out]
